simulink model "VirtualADAPT"  (Simulink 8.9) (continued)
== system VirtualADAPT/VirtualADAPTv1/PowerDistribution/ACLoadBank2 == (continued)
  L2F/1(VinOut0) -> RelayEY275/1(EP2In0)
  RelayEY271/1(EP2Out0) -> L2B/1(InputIn0)
  L2B/1(InputOut0) -> RelayEY271/1(EP2In0)
  RelayEY274/1(EP2Out0) -> L2E/1(InputIn0)
  L2E/1(InputOut0) -> RelayEY274/1(EP2In0)
  ToLoadsIn0/1 -> RelayEY271/2(EP1In0)
  RelayEY271/2(EP1Out0) -> ToLoadsOut0/1
  ToLoadsIn1/1 -> RelayEY272/2(EP1In0)
  RelayEY272/2(EP1Out0) -> ToLoadsOut1/1
  ToLoadsIn2/1 -> RelayEY274/2(EP1In0)
  RelayEY274/2(EP1Out0) -> ToLoadsOut2/1
  ToLoadsIn3/1 -> RelayEY273/2(EP1In0)
  RelayEY273/2(EP1Out0) -> ToLoadsOut3/1
  ToLoadsIn4/1 -> RelayEY275/2(EP1In0)
  RelayEY275/2(EP1Out0) -> ToLoadsOut4/1
  ToLoadsIn5/1 -> RelayEY270/2(EP1In0)
  RelayEY270/2(EP1Out0) -> ToLoadsOut5/1
  L2A/2(FT520) -> FT520/1
  RelayEY275/3(RelayState) -> ESH275A/1
  RelayEY274/3(RelayState) -> ESH274A/1
  RelayEY273/3(RelayState) -> ESH273A/1
  RelayEY272/3(RelayState) -> ESH272A/1
  RelayEY271/3(RelayState) -> ESH271A/1
  RelayEY270/3(RelayState) -> ESH270A/1
  L2F/2(ST516) -> ST516/1
  AmbientTemp/1 -> L2E/2(AmbientTemp), L2B/2(AmbientTemp)
  L2E/4(TE507) -> TE507/1
  L2E/5(TE506) -> TE506/1
  L2E/2(TE505) -> TE505/1
  L2E/3(LT505) -> LT505/1
  L2B/2(TE510) -> TE510/1
  EY273/1 -> RelayEY273/3(SwitchClose)
  EY270/1 -> RelayEY270/3(SwitchClose)
  EY272/1 -> RelayEY272/3(SwitchClose)
  EY274/1 -> RelayEY274/3(SwitchClose)
  EY271/1 -> RelayEY271/3(SwitchClose)
  EY275/1 -> RelayEY275/3(SwitchClose)

== system VirtualADAPT/VirtualADAPTv1/PowerDistribution/CircuitBreakerEY162 ==
  EP2In0 : Inport
  EP1In0 : Inport  Port=2
  CircuitBreakerConnected : Subsystem  mask{Constant=1, FP=Nominal, FT=0, FM=0}
      mask prompts: Constant "Value of Constant:"; FP "Fault Profile:"; FT "Fault Injection Time:"; FM "Fault Magnitude/Slope"
      mask TypeOptions FP: Nominal | Abrupt | Incipient
      mask Display: image(imread('Constant.png','BackgroundColor',[1 1 1]))
  CurrentLimit : Subsystem  mask{Constant=30, FP=Nominal, FT=0, FM=0}
      mask prompts: Constant "Value of Constant:"; FP "Fault Profile:"; FT "Fault Injection Time:"; FM "Fault Magnitude/Slope"
      mask TypeOptions FP: Nominal | Abrupt | Incipient
      mask Display: image(imread('Constant.png','BackgroundColor',[1 1 1]))
  CurrentThrough : Subsystem
      mask Display: image(imread('1.png','BackgroundColor',[1 1 1]))
  DecisionFunction : Subsystem
      mask Display: image(imread('fx.png','BackgroundColor',[1 1 1]))
  Delay : Subsystem
  ParallelConn : Subsystem
      mask Display: image(imread('0.png','BackgroundColor',[1 1 1]))
  EP2Out0 : Outport
  EP1Out0 : Outport  Port=2
  State : Outport  Port=3
  Current : Outport  Port=4
  Voltage : Outport  Port=5
  wires:
  CurrentThrough/1(y(1)) -> ParallelConn/1(u(1))
  ParallelConn/1(y(1)) -> CurrentThrough/1(u(1))
  EP1In0/1 -> CurrentThrough/2(u(2))
  CurrentThrough/2(y(2)) -> EP1Out0/1
  EP2In0/1 -> ParallelConn/2(u(2))
  ParallelConn/2(y(2)) -> EP2Out0/1
  DecisionFunction/1(y(1)) -> ParallelConn/3(u(3)), CurrentThrough/3(u(3))
  CircuitBreakerConnected/1(C) -> CurrentThrough/4(u(4)), ParallelConn/4(u(4))
  Delay/1(Output) -> DecisionFunction/1(u(1))
  CurrentLimit/1(C) -> DecisionFunction/2(u(2))
  CurrentThrough/3(O) -> Delay/1(Input), Current/1
  ParallelConn/3(O) -> Voltage/1
  ParallelConn/4(State) -> State/1

== system VirtualADAPT/VirtualADAPTv1/PowerDistribution/CircuitBreakerEY166 ==
  EP2In0 : Inport
  EP2In1 : Inport  Port=2
  EP2In2 : Inport  Port=3
  EP2In3 : Inport  Port=4
  EP2In4 : Inport  Port=5
  EP2In5 : Inport  Port=6
  EP1In0 : Inport  Port=7
  CircuitBreakerConnected : Subsystem  mask{Constant=1, FP=Nominal, FT=0, FM=0}
      mask prompts: Constant "Value of Constant:"; FP "Fault Profile:"; FT "Fault Injection Time:"; FM "Fault Magnitude/Slope"
      mask TypeOptions FP: Nominal | Abrupt | Incipient
      mask Display: image(imread('Constant.png','BackgroundColor',[1 1 1]))
  CurrentLimit : Subsystem  mask{Constant=30, FP=Nominal, FT=0, FM=0}
      mask prompts: Constant "Value of Constant:"; FP "Fault Profile:"; FT "Fault Injection Time:"; FM "Fault Magnitude/Slope"
      mask TypeOptions FP: Nominal | Abrupt | Incipient
      mask Display: image(imread('Constant.png','BackgroundColor',[1 1 1]))
  CurrentThrough : Subsystem
      mask Display: image(imread('1.png','BackgroundColor',[1 1 1]))
  DecisionFunction : Subsystem
      mask Display: image(imread('fx.png','BackgroundColor',[1 1 1]))
  Delay : Subsystem
  ParallelConn : Subsystem
      mask Display: image(imread('0.png','BackgroundColor',[1 1 1]))
  EP2Out0 : Outport
  EP2Out1 : Outport  Port=2
  EP2Out2 : Outport  Port=3
  EP2Out3 : Outport  Port=4
  EP2Out4 : Outport  Port=5
  EP2Out5 : Outport  Port=6
  EP1Out0 : Outport  Port=7
  State : Outport  Port=8
  Voltage : Outport  Port=9
  Current : Outport  Port=10
  wires:
  CurrentThrough/1(y(1)) -> ParallelConn/1(u(1))
  ParallelConn/1(y(1)) -> CurrentThrough/1(u(1))
  EP1In0/1 -> CurrentThrough/2(u(2))
  CurrentThrough/2(y(2)) -> EP1Out0/1
  EP2In0/1 -> ParallelConn/2(u(2))
  ParallelConn/2(y(2)) -> EP2Out0/1
  EP2In1/1 -> ParallelConn/3(u(3))
  ParallelConn/3(y(3)) -> EP2Out1/1
  EP2In2/1 -> ParallelConn/4(u(4))
  ParallelConn/4(y(4)) -> EP2Out2/1
  EP2In3/1 -> ParallelConn/5(u(5))
  ParallelConn/5(y(5)) -> EP2Out3/1
  EP2In4/1 -> ParallelConn/6(u(6))
  ParallelConn/6(y(6)) -> EP2Out4/1
  EP2In5/1 -> ParallelConn/7(u(7))
  ParallelConn/7(y(7)) -> EP2Out5/1
  DecisionFunction/1(y(1)) -> CurrentThrough/3(u(3)), ParallelConn/8(u(8))
  CircuitBreakerConnected/1(C) -> CurrentThrough/4(u(4)), ParallelConn/9(u(9))
  Delay/1(Output) -> DecisionFunction/1(u(1))
  CurrentLimit/1(C) -> DecisionFunction/2(u(2))
  CurrentThrough/3(O) -> Delay/1(Input), Current/1
  ParallelConn/8(O) -> Voltage/1
  ParallelConn/9(State) -> State/1

== system VirtualADAPT/VirtualADAPTv1/PowerDistribution/CircuitBreakerEY180 ==
  EP2In0 : Inport
  EP2In1 : Inport  Port=2
  EP1In0 : Inport  Port=3
  C : Subsystem  mask{C=2, IC=0, FP=Nominal, FT=0, FM=0}
      mask prompts: C "Value of C:"; IC "Initial Condition:"; FP "Fault Profile:"; FT "Fault Injection Time:"; FM "Fault Magnitude/Slope"
      mask TypeOptions FP: Nominal | Abrupt | Incipient
      mask Display: image(imread('C.png','BackgroundColor',[1 1 1]))
  CircuitBreakerConnected : Subsystem  mask{Constant=1, FP=Nominal, FT=0, FM=0}
      mask prompts: Constant "Value of Constant:"; FP "Fault Profile:"; FT "Fault Injection Time:"; FM "Fault Magnitude/Slope"
      mask TypeOptions FP: Nominal | Abrupt | Incipient
      mask Display: image(imread('Constant.png','BackgroundColor',[1 1 1]))
  CurrentLimit : Subsystem  mask{Constant=30, FP=Nominal, FT=0, FM=0}
      mask prompts: Constant "Value of Constant:"; FP "Fault Profile:"; FT "Fault Injection Time:"; FM "Fault Magnitude/Slope"
      mask TypeOptions FP: Nominal | Abrupt | Incipient
      mask Display: image(imread('Constant.png','BackgroundColor',[1 1 1]))
  CurrentThrough : Subsystem
      mask Display: image(imread('1.png','BackgroundColor',[1 1 1]))
  DecisionFunction : Subsystem
      mask Display: image(imread('fx.png','BackgroundColor',[1 1 1]))
  Delay : Subsystem
  ParallelConn : Subsystem
      mask Display: image(imread('0.png','BackgroundColor',[1 1 1]))
  R : Subsystem  mask{R=0.02, FP=Nominal, FT=0, FM=0}
      mask prompts: R "Value of R:"; FP "Fault Profile:"; FT "Fault Injection Time:"; FM "Fault Magnitude/Slope"
      mask TypeOptions FP: Nominal | Abrupt | Incipient
      mask Display: image(imread('R.png','BackgroundColor',[1 1 1]))
  ZeroJunc : Subsystem
      mask Display: image(imread('0.png','BackgroundColor',[1 1 1]))
  EP2Out0 : Outport
  EP2Out1 : Outport  Port=2
  EP1Out0 : Outport  Port=3
  Current : Outport  Port=4
  Voltage : Outport  Port=5
  State : Outport  Port=6
  wires:
  CurrentThrough/1(y(1)) -> ZeroJunc/1(u(1))
  ZeroJunc/1(y(1)) -> CurrentThrough/1(u(1))
  ZeroJunc/2(y(2)) -> R/1(In)
  R/1(Out) -> ZeroJunc/2(u(2))
  ZeroJunc/3(y(3)) -> C/1(F)
  C/1(E) -> ZeroJunc/3(u(3))
  CurrentThrough/2(y(2)) -> ParallelConn/1(u(1))
  ParallelConn/1(y(1)) -> CurrentThrough/2(u(2))
  EP2In0/1 -> ParallelConn/2(u(2))
  ParallelConn/2(y(2)) -> EP2Out0/1
  EP2In1/1 -> ParallelConn/3(u(3))
  ParallelConn/3(y(3)) -> EP2Out1/1
  EP1In0/1 -> CurrentThrough/3(u(3))
  CurrentThrough/3(y(3)) -> EP1Out0/1
  DecisionFunction/1(y(1)) -> CurrentThrough/4(u(4)), ParallelConn/4(u(4))
  CircuitBreakerConnected/1(C) -> CurrentThrough/5(u(5)), ParallelConn/5(u(5))
  CurrentLimit/1(C) -> DecisionFunction/1(u(1))
  Delay/1(Output) -> DecisionFunction/2(u(2))
  ParallelConn/4(O) -> Voltage/1
  CurrentThrough/4(O) -> Current/1, Delay/1(Input)
  ParallelConn/5(State) -> State/1

== system VirtualADAPT/VirtualADAPTv1/PowerDistribution/CircuitBreakerEY262 ==
  EP2In0 : Inport
  EP1In0 : Inport  Port=2
  CircuitBreakerConnected : Subsystem  mask{Constant=1, FP=Nominal, FT=0, FM=0}
      mask prompts: Constant "Value of Constant:"; FP "Fault Profile:"; FT "Fault Injection Time:"; FM "Fault Magnitude/Slope"
      mask TypeOptions FP: Nominal | Abrupt | Incipient
      mask Display: image(imread('Constant.png','BackgroundColor',[1 1 1]))
  CurrentLimit : Subsystem  mask{Constant=30, FP=Nominal, FT=0, FM=0}
      mask prompts: Constant "Value of Constant:"; FP "Fault Profile:"; FT "Fault Injection Time:"; FM "Fault Magnitude/Slope"
      mask TypeOptions FP: Nominal | Abrupt | Incipient
      mask Display: image(imread('Constant.png','BackgroundColor',[1 1 1]))
  CurrentThrough : Subsystem
      mask Display: image(imread('1.png','BackgroundColor',[1 1 1]))
  DecisionFunction : Subsystem
      mask Display: image(imread('fx.png','BackgroundColor',[1 1 1]))
  Delay : Subsystem
  ParallelConn : Subsystem
      mask Display: image(imread('0.png','BackgroundColor',[1 1 1]))
  EP2Out0 : Outport
  EP1Out0 : Outport  Port=2
  State : Outport  Port=3
  Current : Outport  Port=4
  Voltage : Outport  Port=5
  wires:
  CurrentThrough/1(y(1)) -> ParallelConn/1(u(1))
  ParallelConn/1(y(1)) -> CurrentThrough/1(u(1))
  EP1In0/1 -> CurrentThrough/2(u(2))
  CurrentThrough/2(y(2)) -> EP1Out0/1
  EP2In0/1 -> ParallelConn/2(u(2))
  ParallelConn/2(y(2)) -> EP2Out0/1
  DecisionFunction/1(y(1)) -> CurrentThrough/3(u(3)), ParallelConn/3(u(3))
  CircuitBreakerConnected/1(C) -> CurrentThrough/4(u(4)), ParallelConn/4(u(4))
  CurrentLimit/1(C) -> DecisionFunction/1(u(1))
  Delay/1(Output) -> DecisionFunction/2(u(2))
  ParallelConn/3(O) -> Voltage/1
  CurrentThrough/3(O) -> Current/1, Delay/1(Input)
  ParallelConn/4(State) -> State/1

== system VirtualADAPT/VirtualADAPTv1/PowerDistribution/CircuitBreakerEY266 ==
  EP2In0 : Inport
  EP2In1 : Inport  Port=2
  EP2In2 : Inport  Port=3
  EP2In3 : Inport  Port=4
  EP2In4 : Inport  Port=5
  EP2In5 : Inport  Port=6
  EP1In0 : Inport  Port=7
  CircuitBreakerConnected : Subsystem  mask{Constant=1, FP=Nominal, FT=0, FM=0}
      mask prompts: Constant "Value of Constant:"; FP "Fault Profile:"; FT "Fault Injection Time:"; FM "Fault Magnitude/Slope"
      mask TypeOptions FP: Nominal | Abrupt | Incipient
      mask Display: image(imread('Constant.png','BackgroundColor',[1 1 1]))
  CurrentLimit : Subsystem  mask{Constant=30, FP=Nominal, FT=0, FM=0}
      mask prompts: Constant "Value of Constant:"; FP "Fault Profile:"; FT "Fault Injection Time:"; FM "Fault Magnitude/Slope"
      mask TypeOptions FP: Nominal | Abrupt | Incipient
      mask Display: image(imread('Constant.png','BackgroundColor',[1 1 1]))
  CurrentThrough : Subsystem
      mask Display: image(imread('1.png','BackgroundColor',[1 1 1]))
  DecisionFunction : Subsystem
      mask Display: image(imread('fx.png','BackgroundColor',[1 1 1]))
  Delay : Subsystem
  ParallelConn : Subsystem
      mask Display: image(imread('0.png','BackgroundColor',[1 1 1]))
  EP2Out0 : Outport
  EP2Out1 : Outport  Port=2
  EP2Out2 : Outport  Port=3
  EP2Out3 : Outport  Port=4
  EP2Out4 : Outport  Port=5
  EP2Out5 : Outport  Port=6
  EP1Out0 : Outport  Port=7
  Current : Outport  Port=8
  Voltage : Outport  Port=9
  State : Outport  Port=10
  wires:
  CurrentThrough/1(y(1)) -> ParallelConn/1(u(1))
  ParallelConn/1(y(1)) -> CurrentThrough/1(u(1))
  EP1In0/1 -> CurrentThrough/2(u(2))
  CurrentThrough/2(y(2)) -> EP1Out0/1
  EP2In0/1 -> ParallelConn/2(u(2))
  ParallelConn/2(y(2)) -> EP2Out0/1
  EP2In1/1 -> ParallelConn/3(u(3))
  ParallelConn/3(y(3)) -> EP2Out1/1
  EP2In2/1 -> ParallelConn/4(u(4))
  ParallelConn/4(y(4)) -> EP2Out2/1
  EP2In3/1 -> ParallelConn/5(u(5))
  ParallelConn/5(y(5)) -> EP2Out3/1
  EP2In4/1 -> ParallelConn/6(u(6))
  ParallelConn/6(y(6)) -> EP2Out4/1
  EP2In5/1 -> ParallelConn/7(u(7))
  ParallelConn/7(y(7)) -> EP2Out5/1
  DecisionFunction/1(y(1)) -> CurrentThrough/3(u(3)), ParallelConn/8(u(8))
  CircuitBreakerConnected/1(C) -> CurrentThrough/4(u(4)), ParallelConn/9(u(9))
  Delay/1(Output) -> DecisionFunction/1(u(1))
  CurrentLimit/1(C) -> DecisionFunction/2(u(2))
  CurrentThrough/3(O) -> Delay/1(Input), Current/1
  ParallelConn/8(O) -> Voltage/1
  ParallelConn/9(State) -> State/1

== system VirtualADAPT/VirtualADAPTv1/PowerDistribution/CircuitBreakerEY280 ==
  EP2In0 : Inport
  EP2In1 : Inport  Port=2
  EP1In0 : Inport  Port=3
  C : Subsystem  mask{C=2, IC=0, FP=Nominal, FT=0, FM=0}
      mask prompts: C "Value of C:"; IC "Initial Condition:"; FP "Fault Profile:"; FT "Fault Injection Time:"; FM "Fault Magnitude/Slope"
      mask TypeOptions FP: Nominal | Abrupt | Incipient
      mask Display: image(imread('C.png','BackgroundColor',[1 1 1]))
  CircuitBreakerConnected : Subsystem  mask{Constant=1, FP=Nominal, FT=0, FM=0}
      mask prompts: Constant "Value of Constant:"; FP "Fault Profile:"; FT "Fault Injection Time:"; FM "Fault Magnitude/Slope"
      mask TypeOptions FP: Nominal | Abrupt | Incipient
      mask Display: image(imread('Constant.png','BackgroundColor',[1 1 1]))
  CurrentLimit : Subsystem  mask{Constant=30, FP=Nominal, FT=0, FM=0}
      mask prompts: Constant "Value of Constant:"; FP "Fault Profile:"; FT "Fault Injection Time:"; FM "Fault Magnitude/Slope"
      mask TypeOptions FP: Nominal | Abrupt | Incipient
      mask Display: image(imread('Constant.png','BackgroundColor',[1 1 1]))
  CurrentThrough : Subsystem
      mask Display: image(imread('1.png','BackgroundColor',[1 1 1]))
  DecisionFunction : Subsystem
      mask Display: image(imread('fx.png','BackgroundColor',[1 1 1]))
  Delay : Subsystem
  ParallelConn : Subsystem
      mask Display: image(imread('0.png','BackgroundColor',[1 1 1]))
  R : Subsystem  mask{R=0.02, FP=Nominal, FT=0, FM=0}
      mask prompts: R "Value of R:"; FP "Fault Profile:"; FT "Fault Injection Time:"; FM "Fault Magnitude/Slope"
      mask TypeOptions FP: Nominal | Abrupt | Incipient
      mask Display: image(imread('R.png','BackgroundColor',[1 1 1]))
  ZeroJunc : Subsystem
      mask Display: image(imread('0.png','BackgroundColor',[1 1 1]))
  EP2Out0 : Outport
  EP2Out1 : Outport  Port=2
  EP1Out0 : Outport  Port=3
  Current : Outport  Port=4
  Voltage : Outport  Port=5
  State : Outport  Port=6
  wires:
  CurrentThrough/1(y(1)) -> ZeroJunc/1(u(1))
  ZeroJunc/1(y(1)) -> CurrentThrough/1(u(1))
  ZeroJunc/2(y(2)) -> R/1(In)
  R/1(Out) -> ZeroJunc/2(u(2))
  ZeroJunc/3(y(3)) -> C/1(F)
  C/1(E) -> ZeroJunc/3(u(3))
  CurrentThrough/2(y(2)) -> ParallelConn/1(u(1))
  ParallelConn/1(y(1)) -> CurrentThrough/2(u(2))
  EP2In0/1 -> ParallelConn/2(u(2))
  ParallelConn/2(y(2)) -> EP2Out0/1
  EP2In1/1 -> ParallelConn/3(u(3))
  ParallelConn/3(y(3)) -> EP2Out1/1
  EP1In0/1 -> CurrentThrough/3(u(3))
  CurrentThrough/3(y(3)) -> EP1Out0/1
  DecisionFunction/1(y(1)) -> CurrentThrough/4(u(4)), ParallelConn/4(u(4))
  CircuitBreakerConnected/1(C) -> CurrentThrough/5(u(5)), ParallelConn/5(u(5))
  CurrentLimit/1(C) -> DecisionFunction/1(u(1))
  Delay/1(Output) -> DecisionFunction/2(u(2))
  ParallelConn/4(O) -> Voltage/1
  CurrentThrough/4(O) -> Current/1, Delay/1(Input)
  ParallelConn/5(State) -> State/1

== system VirtualADAPT/VirtualADAPTv1/PowerDistribution/DCLoadBank1 ==
  ToLoadsIn0 : Inport
  ToLoadsIn1 : Inport  Port=2
  EY184 : Inport  Port=3
  EY183 : Inport  Port=4
  L1G : Subsystem
  L1H : Subsystem
  RelayEY183 : Subsystem
  RelayEY184 : Subsystem
  ToLoadsOut0 : Outport
  ToLoadsOut1 : Outport  Port=2
  ESH184A : Outport  Port=3
  ESH183A : Outport  Port=4
  wires:
  RelayEY183/1(EP2Out0) -> L1G/1(EnergyPortIn0)
  L1G/1(EnergyPortOut0) -> RelayEY183/1(EP2In0)
  RelayEY184/1(EP2Out0) -> L1H/1(EPIn0)
  L1H/1(EPOut0) -> RelayEY184/1(EP2In0)
  ToLoadsIn0/1 -> RelayEY183/2(EP1In0)
  RelayEY183/2(EP1Out0) -> ToLoadsOut0/1
  ToLoadsIn1/1 -> RelayEY184/2(EP1In0)
  RelayEY184/2(EP1Out0) -> ToLoadsOut1/1
  RelayEY184/3(RelayState) -> ESH184A/1
  RelayEY183/3(RelayState) -> ESH183A/1
  EY183/1 -> RelayEY183/3(SwitchClose)
  EY184/1 -> RelayEY184/3(SwitchClose)

== system VirtualADAPT/VirtualADAPTv1/PowerDistribution/DCLoadBank2 ==
  ToLoadsIn0 : Inport
  ToLoadsIn1 : Inport  Port=2
  EY284 : Inport  Port=3
  EY283 : Inport  Port=4
  Load2G : Subsystem
  Load2H : Subsystem
  RelayEY283 : Subsystem
  RelayEY284 : Subsystem
  ToLoadsOut0 : Outport
  ToLoadsOut1 : Outport  Port=2
  ESH284A : Outport  Port=3
  ESH283A : Outport  Port=4
  wires:
  RelayEY284/2(EP2Out0) -> Load2H/1(EnergyPortIn0)
  Load2H/1(EnergyPortOut0) -> RelayEY284/2(EP2In0)
  ToLoadsIn0/1 -> RelayEY284/1(EP1In0)
  RelayEY284/1(EP1Out0) -> ToLoadsOut0/1
  RelayEY283/1(EP2Out0) -> Load2G/1(EPIn0)
  Load2G/1(EPOut0) -> RelayEY283/1(EP2In0)
  ToLoadsIn1/1 -> RelayEY283/2(EP1In0)
  RelayEY283/2(EP1Out0) -> ToLoadsOut1/1
  RelayEY283/3(RelayState) -> ESH283A/1
  RelayEY284/3(RelayState) -> ESH284A/1
  EY284/1 -> RelayEY284/3(SwitchClose)
  EY283/1 -> RelayEY283/3(SwitchClose)

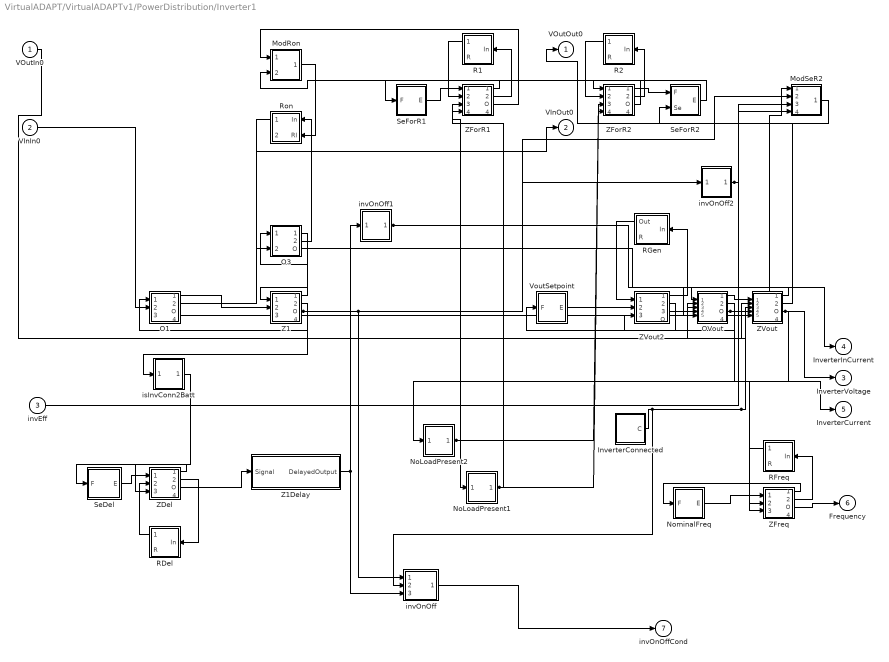
== system VirtualADAPT/VirtualADAPTv1/PowerDistribution/Inverter1 ==
  VOutIn0 : Inport
  VInIn0 : Inport  Port=2
  invEff : Inport  Port=3
  InverterConnected : Subsystem  mask{Constant=1, FP=Nominal, FT=0, FM=0}
      mask prompts: Constant "Value of Constant:"; FP "Fault Profile:"; FT "Fault Injection Time:"; FM "Fault Magnitude/Slope"
      mask TypeOptions FP: Nominal | Abrupt | Incipient
      mask Display: image(imread('Constant.png','BackgroundColor',[1 1 1]))
  ModRon : Subsystem
      mask Display: image(imread('fx.png','BackgroundColor',[1 1 1]))
  ModSeR2 : Subsystem
      mask Display: image(imread('fx.png','BackgroundColor',[1 1 1]))
  NoLoadPresent1 : Subsystem
      mask Display: image(imread('fx.png','BackgroundColor',[1 1 1]))
  NoLoadPresent2 : Subsystem
      mask Display: image(imread('fx.png','BackgroundColor',[1 1 1]))
  NominalFreq : Subsystem  mask{Se=60, FP=Nominal, FT=0, FM=0}
      mask prompts: Se "Value of Se:"; FP "Fault Profile:"; FT "Fault Injection Time:"; FM "Fault Magnitude/Slope/Bias/StuckAtValue"
      mask TypeOptions FP: Nominal | Abrupt | Incipient | Bias | StuckAt
      mask Display: image(imread('Se.png','BackgroundColor',[1 1 1]))
  O1 : Subsystem
      mask Display: image(imread('1.png','BackgroundColor',[1 1 1]))
  O3 : Subsystem
      mask Display: image(imread('1.png','BackgroundColor',[1 1 1]))
  OVout : Subsystem
      mask Display: image(imread('1.png','BackgroundColor',[1 1 1]))
  R1 : Subsystem  mask{R=1, FP=Nominal, FT=0, FM=0}
      mask prompts: R "Value of R:"; FP "Fault Profile:"; FT "Fault Injection Time:"; FM "Fault Magnitude/Slope"
      mask TypeOptions FP: Nominal | Abrupt | Incipient
      mask Display: image(imread('R.png','BackgroundColor',[1 1 1]))
  R2 : Subsystem  mask{R=1, FP=Nominal, FT=0, FM=0}
      mask prompts: R "Value of R:"; FP "Fault Profile:"; FT "Fault Injection Time:"; FM "Fault Magnitude/Slope"
      mask TypeOptions FP: Nominal | Abrupt | Incipient
      mask Display: image(imread('R.png','BackgroundColor',[1 1 1]))
  RDel : Subsystem  mask{R=1, FP=Nominal, FT=0, FM=0}
      mask prompts: R "Value of R:"; FP "Fault Profile:"; FT "Fault Injection Time:"; FM "Fault Magnitude/Slope"
      mask TypeOptions FP: Nominal | Abrupt | Incipient
      mask Display: image(imread('R.png','BackgroundColor',[1 1 1]))
  RFreq : Subsystem  mask{R=1, FP=Nominal, FT=0, FM=0}
      mask prompts: R "Value of R:"; FP "Fault Profile:"; FT "Fault Injection Time:"; FM "Fault Magnitude/Slope"
      mask TypeOptions FP: Nominal | Abrupt | Incipient
      mask Display: image(imread('R.png','BackgroundColor',[1 1 1]))
  RGen : Subsystem  mask{R=1, FP=Nominal, FT=0, FM=0}
      mask prompts: R "Value of R:"; FP "Fault Profile:"; FT "Fault Injection Time:"; FM "Fault Magnitude/Slope"
      mask TypeOptions FP: Nominal | Abrupt | Incipient
      mask Display: image(imread('R.png','BackgroundColor',[1 1 1]))
  Ron : Subsystem  mask{FP=Nominal, FT=0, FM=0}
      mask prompts: FP "Fault Profile:"; FT "Fault Injection Time:"; FM "Fault Magnitude/Slope"
      mask TypeOptions FP: Nominal | Abrupt | Incipient
      mask Display: image(imread('MR.png','BackgroundColor',[1 1 1]))
  SeDel : Subsystem  mask{Se=1, FP=Nominal, FT=0, FM=0}
      mask prompts: Se "Value of Se:"; FP "Fault Profile:"; FT "Fault Injection Time:"; FM "Fault Magnitude/Slope/Bias/StuckAtValue"
      mask TypeOptions FP: Nominal | Abrupt | Incipient | Bias | StuckAt
      mask Display: image(imread('Se.png','BackgroundColor',[1 1 1]))
  SeForR1 : Subsystem  mask{Se=23, FP=Nominal, FT=0, FM=0}
      mask prompts: Se "Value of Se:"; FP "Fault Profile:"; FT "Fault Injection Time:"; FM "Fault Magnitude/Slope/Bias/StuckAtValue"
      mask TypeOptions FP: Nominal | Abrupt | Incipient | Bias | StuckAt
      mask Display: image(imread('Se.png','BackgroundColor',[1 1 1]))
  SeForR2 : Subsystem  mask{FP=Nominal, FT=0, FM=0}
      mask prompts: FP "Fault Profile:"; FT "Fault Injection Time:"; FM "Fault Magnitude/Slope/Bias/StuckAtValue"
      mask TypeOptions FP: Nominal | Abrupt | Incipient | Bias | StuckAt
      mask Display: image(imread('MSe.png','BackgroundColor',[1 1 1]))
  VoutSetpoint : Subsystem  mask{Se=120.5, FP=Nominal, FT=0, FM=0}
      mask prompts: Se "Value of Se:"; FP "Fault Profile:"; FT "Fault Injection Time:"; FM "Fault Magnitude/Slope/Bias/StuckAtValue"
      mask TypeOptions FP: Nominal | Abrupt | Incipient | Bias | StuckAt
      mask Display: image(imread('Se.png','BackgroundColor',[1 1 1]))
  Z1 : Subsystem
      mask Display: image(imread('0.png','BackgroundColor',[1 1 1]))
  Z1Delay : Subsystem
  ZDel : Subsystem
      mask Display: image(imread('0.png','BackgroundColor',[1 1 1]))
  ZForR1 : Subsystem
      mask Display: image(imread('0.png','BackgroundColor',[1 1 1]))
  ZForR2 : Subsystem
      mask Display: image(imread('0.png','BackgroundColor',[1 1 1]))
  ZFreq : Subsystem
      mask Display: image(imread('0.png','BackgroundColor',[1 1 1]))
  ZVout : Subsystem
      mask Display: image(imread('0.png','BackgroundColor',[1 1 1]))
  ZVout2 : Subsystem
      mask Display: image(imread('0.png','BackgroundColor',[1 1 1]))
  invOnOff : Subsystem
      mask Display: image(imread('fx.png','BackgroundColor',[1 1 1]))
  invOnOff1 : Subsystem
      mask Display: image(imread('fx.png','BackgroundColor',[1 1 1]))
  invOnOff2 : Subsystem
      mask Display: image(imread('fx.png','BackgroundColor',[1 1 1]))
  isInvConn2Batt : Subsystem
      mask Display: image(imread('fx.png','BackgroundColor',[1 1 1]))
  VOutOut0 : Outport
  VInOut0 : Outport  Port=2
  InverterVoltage : Outport  Port=3
  InverterInCurrent : Outport  Port=4
  InverterCurrent : Outport  Port=5
  Frequency : Outport  Port=6
  invOnOffCond : Outport  Port=7
  wires:
  ZVout2/1(y(1)) -> RGen/1(In)
  RGen/1(Out) -> ZVout2/1(u(1))
  SeDel/1(E) -> ZDel/1(u(1))
  ZDel/1(y(1)) -> SeDel/1(F)
  ZDel/2(y(2)) -> RDel/1(In)
  RDel/1(Out) -> ZDel/2(u(2))
  VoutSetpoint/1(E) -> ZVout2/2(u(2))
  ZVout2/2(y(2)) -> VoutSetpoint/1(F)
  Z1/1(y(1)) -> O3/1(u(1))
  O3/1(y(1)) -> Z1/1(u(1))
  OVout/1(y(1)) -> ZVout/1(u(1))
  ZVout/1(y(1)) -> OVout/1(u(1))
  O1/1(y(1)) -> Z1/2(u(2))
  Z1/2(y(2)) -> O1/1(u(1))
  ZVout2/3(y(3)) -> OVout/2(u(2))
  OVout/2(y(2)) -> ZVout2/3(u(3))
  SeForR1/1(E) -> ZForR1/1(u(1))
  ZForR1/1(y(1)) -> SeForR1/1(F)
  SeForR2/1(E) -> ZForR2/1(u(1))
  ZForR2/1(y(1)) -> SeForR2/1(F)
  O3/2(y(2)) -> Ron/1(In)
  Ron/1(Out) -> O3/2(u(2))
  ZForR1/2(y(2)) -> R1/1(In)
  R1/1(Out) -> ZForR1/2(u(2))
  ZForR2/2(y(2)) -> R2/1(In)
  R2/1(Out) -> ZForR2/2(u(2))
  NominalFreq/1(E) -> ZFreq/1(u(1))
  ZFreq/1(y(1)) -> NominalFreq/1(F)
  ZFreq/2(y(2)) -> RFreq/1(In)
  RFreq/1(Out) -> ZFreq/2(u(2))
  VInIn0/1 -> O1/2(u(2))
  O1/2(y(2)) -> VInOut0/1
  VOutIn0/1 -> ZVout/2(u(2))
  ZVout/2(y(2)) -> VOutOut0/1
  ModRon/1(y(1)) -> Ron/2(RI)
  ModSeR2/1(y(1)) -> SeForR2/2(Se)
  NoLoadPresent2/1(y(1)) -> ZForR2/3(u(3)), ZForR1/3(u(3))
  invOnOff2/1(y(1)) -> ZVout/4(u(4)), OVout/4(u(4))
  invOnOff1/1(y(1)) -> ZFreq/3(u(3)), OVout/5(u(5)), ZVout/5(u(5))
  isInvConn2Batt/1(y(1)) -> ZDel/3(u(3))
  NoLoadPresent1/1(y(1)) -> ZForR1/4(u(4)), ZForR2/4(u(4))
  ZVout/3(O) -> NoLoadPresent2/1(u(1)), ModSeR2/1(u(1)), InverterVoltage/1
  Z1/3(O) -> invOnOff2/1(u(1)), isInvConn2Batt/1(u(1)), ModSeR2/2(u(2)), invOnOff/1(u(1))
  Z1Delay/1(DelayedOutput) -> invOnOff1/1(u(1)), invOnOff/3(u(3))
  OVout/3(O) -> NoLoadPresent1/1(u(1)), ModSeR2/3(u(3)), InverterCurrent/1
  ZForR1/3(O) -> ModRon/1(u(1))
  ZForR2/3(O) -> ModRon/2(u(2))
  O3/3(O) -> InverterInCurrent/1
  ZDel/3(O) -> Z1Delay/1(Signal)
  ZFreq/3(O) -> Frequency/1
  invOnOff/1(y(1)) -> invOnOffCond/1
  InverterConnected/1(C) -> ZVout/3(u(3)), OVout/3(u(3)), O1/3(u(3)), Z1/3(u(3)), invOnOff/2(u(2))
  invEff/1 -> ModSeR2/4(u(4))
